simulink model "AcasXuClosedLoop_all_comp"  (Simulink 10.0)
settings: Solver=VariableStepAuto  StartTime=0.0  StopTime=20  RelTol=1e-3
libraries: neural x14040, simulink x2
(part 27 of 534)

== system AcasXuClosedLoop_all_comp/networks/Networks_m_6/AcasXu_4_6/Layer 3 ==
  "a{2} " : Inport  PortDimensions=50
      mask Display: e = 0.06;c1 = [120 230 180]/255;c2 = ([120 230 180]+0)/(256+128);c3 = ([120 230 180]+128)/(256+128);patch([0 1 1 0],[0 0 1 1],c1);patch([0 1 1 1-e 1-e 0+e],[0 0 1 1-e 0+e 0+e],c2);patch([0 0 1 1-e 0+e 0+e],[0 1 1 1-e 1-e 0+e],c3);plot([0 1 1 0 0],[0 0 1 1 0]);
  Delays 1 : Subsystem
      mask Display: e = 0.06;c1 = [120 150 230]/255;c2 = ([120 150 230]+0)/383;c3 = ([120 150 230]+128)/383;patch([0 1 1 0],[0 0 1 1],c1);patch([0 1 1 1-e 1-e 0+e],[0 0 1 1-e 0+e 0+e],c2);patch([0 0 1 1-e 0+e 0+e],[0 1 1 1-e 1-e 0+e],c3);plot([0 1 1 0 0],[0 0 1 1 0]);disp('0');
  "LW{3,2}" : Subsystem
      mask Display: e = 0.06;c1 = [120 150 230]/255;c2 = ([120 150 230]+0)/383;c3 = ([120 150 230]+128)/383;patch([0 1 1 0],[0 0 1 1],c1);patch([0 1 1 1-e 1-e 0+e],[0 0 1 1-e 0+e 0+e],c2);patch([0 0 1 1-e 0+e 0+e],[0 1 1 1-e 1-e 0+e],c3);plot([0 1 1 0 0],[0 0 1 1 0]);disp('W');
  "b{3}" : Constant  Value=[1.25236999999999998323119143606163561344146728515625;-1.82186000000000003495870259939692914485931396484375;0.70690799999999998082245156183489598333835601806640625;0.67740699999999998137667489572777412831783294677734375;0.003204180000000000048954174047821652493439614772796630859375;-1.4122500000000000053290705182007513940334320068359375;-0.1815900000000000014122036873231991194188594818115234375;-2.066549999999999887023705014144070446491241455078125;-0.5674580000000000179483095053001306951045989990234375;-0.235665999999999986602716717243310995399951934814453125;-0.3740229999999999943582906780648045241832733154296875;-0.033654700000000002668709697672966285608708858489990234375;0.60203799999999996206412333776825107634067535400390625;-0.89405599999999996185806594439782202243804931640625;-1.5173799999999999510436055061290971934795379638671875;-0.09026889999999999913971038267845869995653629302978515625;-0.420567999999999997395860873439232818782329559326171875;-0.931707999999999980644815877894870936870574951171875;-0.06573710000000000663877841589055606164038181304931640625;-0.339210000000000011510792319313623011112213134765625;-0.75268800000000002370370566495694220066070556640625;-1.114430000000000031690206014900468289852142333984375;-0.5772559999999999913455894784419797360897064208984375;0.357165000000000010249578963339445181190967559814453125;-1.9052100000000000701305680195218883454799652099609375;0.109812999999999993949728605002746917307376861572265625;2.244800000000000128608235172578133642673492431640625;-2.8195899999999998186694938340224325656890869140625;-0.2012519999999999864570554564124904572963714599609375;-1.2382999999999999563016217507538385689258575439453125;0.1436860000000000081588069633653503842651844024658203125;0.11354699999999999515676307737521710805594921112060546875;-0.571150999999999964273911245982162654399871826171875;-0.53546199999999999352695567722548730671405792236328125;-1.1326199999999999601385525238583795726299285888671875;0.90043499999999998539834678012994118034839630126953125;0.56335199999999996389732359602930955588817596435546875;-0.2246470000000000133422162207352812401950359344482421875;-1.126980000000000092796881290269084274768829345703125;-0.93247400000000002506794771761633455753326416015625;-1.440020000000000077733375292154960334300994873046875;-0.73937600000000003319655661471188068389892578125;-0.835104999999999986215470926254056394100189208984375;0.3871350000000000068922645368729718029499053955078125;-1.428290000000000059543481256696395576000213623046875;-0.748561000000000031917579690343700349330902099609375;-0.296891999999999989245935694270883686840534210205078125;-1.4761800000000000476774175695027224719524383544921875;0.352663000000000004252598273524199612438678741455078125;0.5822629999999999750315282653900794684886932373046875]
      mask Display: e = 0.06;c1 = [120 150 230]/255;c2 = ([120 150 230]+0)/383;c3 = ([120 150 230]+128)/383;patch([0 1 1 0],[0 0 1 1],c1);patch([0 1 1 1-e 1-e 0+e],[0 0 1 1-e 0+e 0+e],c2);patch([0 0 1 1-e 0+e 0+e],[0 1 1 1-e 1-e 0+e],c3);plot([0 1 1 0 0],[0 0 1 1 0]);disp('b');
  netsum : Sum  Inputs=2  SaturateOnIntegerOverflow=on  InputSameDT=on  OutDataTypeStr=Inherit: Same as first input
      mask Display: e = 0.06;c1 = [120 150 230]/255;c2 = ([120 150 230]+0)/383;c3 = ([120 150 230]+128)/383;patch([0 1 1 0],[0 0 1 1],c1);patch([0 1 1 1-e 1-e 0+e],[0 0 1 1-e 0+e 0+e],c2);patch([0 0 1 1-e 0+e 0+e],[0 1 1 1-e 1-e 0+e],c3);plot([0 1 1 0 0],[0 0 1 1 0]);disp('+');
  poslin : neural/Transfer Functions/poslin
      settings: SourceProductName=Deep Learning Toolbox  SourceProductBaseCode=NN
  "a{3}" : Outport  InitialOutput=[0;0;0;0;0;0;0;0;0;0;0;0;0;0;0;0;0;0;0;0;0;0;0;0;0;0;0;0;0;0;0;0;0;0;0;0;0;0;0;0;0;0;0;0;0;0;0;0;0;0]
      mask Display: e = 0.06;c1 = [120 230 180]/255;c2 = ([120 230 180]+0)/(256+128);c3 = ([120 230 180]+128)/(256+128);patch([0 1 1 0],[0 0 1 1],c1);patch([0 1 1 1-e 1-e 0+e],[0 0 1 1-e 0+e 0+e],c2);patch([0 0 1 1-e 0+e 0+e],[0 1 1 1-e 1-e 0+e],c3);plot([0 1 1 0 0],[0 0 1 1 0]);
  wires:
  poslin/1 -> "a{3}"/1
  netsum/1 -> poslin/1
  "b{3}"/1 -> netsum/2
  "LW{3,2}"/1("lz{3,2}") -> netsum/1
  Delays 1/1("pd{3,2}") -> "LW{3,2}"/1("ad{3,2}")
  "a{2} "/1 -> Delays 1/1("p{3}")

== system AcasXuClosedLoop_all_comp/networks/Networks_m_6/AcasXu_4_6/Layer 4 ==
  "a{3} " : Inport  PortDimensions=50
      mask Display: e = 0.06;c1 = [120 230 180]/255;c2 = ([120 230 180]+0)/(256+128);c3 = ([120 230 180]+128)/(256+128);patch([0 1 1 0],[0 0 1 1],c1);patch([0 1 1 1-e 1-e 0+e],[0 0 1 1-e 0+e 0+e],c2);patch([0 0 1 1-e 0+e 0+e],[0 1 1 1-e 1-e 0+e],c3);plot([0 1 1 0 0],[0 0 1 1 0]);
  Delays 1 : Subsystem
      mask Display: e = 0.06;c1 = [120 150 230]/255;c2 = ([120 150 230]+0)/383;c3 = ([120 150 230]+128)/383;patch([0 1 1 0],[0 0 1 1],c1);patch([0 1 1 1-e 1-e 0+e],[0 0 1 1-e 0+e 0+e],c2);patch([0 0 1 1-e 0+e 0+e],[0 1 1 1-e 1-e 0+e],c3);plot([0 1 1 0 0],[0 0 1 1 0]);disp('0');
  "LW{4,3}" : Subsystem
      mask Display: e = 0.06;c1 = [120 150 230]/255;c2 = ([120 150 230]+0)/383;c3 = ([120 150 230]+128)/383;patch([0 1 1 0],[0 0 1 1],c1);patch([0 1 1 1-e 1-e 0+e],[0 0 1 1-e 0+e 0+e],c2);patch([0 0 1 1-e 0+e 0+e],[0 1 1 1-e 1-e 0+e],c3);plot([0 1 1 0 0],[0 0 1 1 0]);disp('W');
  "b{4}" : Constant  Value=[-3.334090000000000220126139538479037582874298095703125;-0.76609099999999996644106659005046822130680084228515625;-0.047776800000000001211741817996880854479968547821044921875;1.966110000000000024300561562995426356792449951171875;-1.510219999999999895834434937569312751293182373046875;-2.430120000000000057838178690872155129909515380859375;-1.6310599999999999543121020906255580484867095947265625;0.365655000000000007798206524967099539935588836669921875;1.6739200000000000745359329812345094978809356689453125;-0.32896599999999998065192130525247193872928619384765625;2.846200000000000063238303482648916542530059814453125;-0.67345800000000000107291953099775128066539764404296875;-0.61934500000000003439026841078884899616241455078125;-2.510380000000000055848659030743874609470367431640625;-0.0987428999999999945647033428031136281788349151611328125;-3.1409099999999998686917024315334856510162353515625;0.2509770000000000056417093219351954758167266845703125;0.9941429999999999989057641869294457137584686279296875;-1.0512200000000000432720526077901013195514678955078125;-1.0510900000000000797939492258592508733272552490234375;-0.1089090000000000058033577943206182681024074554443359375;-2.9356700000000000017053025658242404460906982421875;-0.15021899999999999142374917937559075653553009033203125;-2.948770000000000113260512080159969627857208251953125;0.08955470000000000097006846999647677876055240631103515625;1.2601299999999999723598875789321027696132659912109375;2.14989000000000007872813512221910059452056884765625;0.5562270000000000269579913947382010519504547119140625;-1.414260000000000072617467594682238996028900146484375;-2.142910000000000092512664195965044200420379638671875;0.08335019999999999928963489992383983917534351348876953125;-0.452707000000000026052049406644073314964771270751953125;0.73034699999999996844479710489395074546337127685546875;0.386303000000000007485567721232655458152294158935546875;0.2329029999999999989146459711264469660818576812744140625;-1.114360000000000017195134205394424498081207275390625;-4.6393000000000004234834705130197107791900634765625;0.265996999999999983455012397826067171990871429443359375;0.037446699999999999430855268656159751117229461669921875;-0.84933800000000003738165332833887077867984771728515625;0.08166610000000000535447242100417497567832469940185546875;-0.407220999999999999641175918441149406135082244873046875;-1.1031500000000000749622586226905696094036102294921875;-0.765932999999999974960473991814069449901580810546875;1.071849999999999969446662362315692007541656494140625;0.6187460000000000182041048901737667620182037353515625;-1.0859799999999999453592636200482957065105438232421875;0.0642923999999999995491606341602164320647716522216796875;0.260197999999999984854781587273464538156986236572265625;-4.03394999999999992468247000942938029766082763671875]
      mask Display: e = 0.06;c1 = [120 150 230]/255;c2 = ([120 150 230]+0)/383;c3 = ([120 150 230]+128)/383;patch([0 1 1 0],[0 0 1 1],c1);patch([0 1 1 1-e 1-e 0+e],[0 0 1 1-e 0+e 0+e],c2);patch([0 0 1 1-e 0+e 0+e],[0 1 1 1-e 1-e 0+e],c3);plot([0 1 1 0 0],[0 0 1 1 0]);disp('b');
  netsum : Sum  Inputs=2  SaturateOnIntegerOverflow=on  InputSameDT=on  OutDataTypeStr=Inherit: Same as first input
      mask Display: e = 0.06;c1 = [120 150 230]/255;c2 = ([120 150 230]+0)/383;c3 = ([120 150 230]+128)/383;patch([0 1 1 0],[0 0 1 1],c1);patch([0 1 1 1-e 1-e 0+e],[0 0 1 1-e 0+e 0+e],c2);patch([0 0 1 1-e 0+e 0+e],[0 1 1 1-e 1-e 0+e],c3);plot([0 1 1 0 0],[0 0 1 1 0]);disp('+');
  poslin : neural/Transfer Functions/poslin
      settings: SourceProductName=Deep Learning Toolbox  SourceProductBaseCode=NN
  "a{4}" : Outport  InitialOutput=[0;0;0;0;0;0;0;0;0;0;0;0;0;0;0;0;0;0;0;0;0;0;0;0;0;0;0;0;0;0;0;0;0;0;0;0;0;0;0;0;0;0;0;0;0;0;0;0;0;0]
      mask Display: e = 0.06;c1 = [120 230 180]/255;c2 = ([120 230 180]+0)/(256+128);c3 = ([120 230 180]+128)/(256+128);patch([0 1 1 0],[0 0 1 1],c1);patch([0 1 1 1-e 1-e 0+e],[0 0 1 1-e 0+e 0+e],c2);patch([0 0 1 1-e 0+e 0+e],[0 1 1 1-e 1-e 0+e],c3);plot([0 1 1 0 0],[0 0 1 1 0]);
  wires:
  poslin/1 -> "a{4}"/1
  netsum/1 -> poslin/1
  "b{4}"/1 -> netsum/2
  "LW{4,3}"/1("lz{4,3}") -> netsum/1
  Delays 1/1("pd{4,3}") -> "LW{4,3}"/1("ad{4,3}")
  "a{3} "/1 -> Delays 1/1("p{4}")

== system AcasXuClosedLoop_all_comp/networks/Networks_m_6/AcasXu_4_6/Layer 5 ==
  "a{4} " : Inport  PortDimensions=50
      mask Display: e = 0.06;c1 = [120 230 180]/255;c2 = ([120 230 180]+0)/(256+128);c3 = ([120 230 180]+128)/(256+128);patch([0 1 1 0],[0 0 1 1],c1);patch([0 1 1 1-e 1-e 0+e],[0 0 1 1-e 0+e 0+e],c2);patch([0 0 1 1-e 0+e 0+e],[0 1 1 1-e 1-e 0+e],c3);plot([0 1 1 0 0],[0 0 1 1 0]);
  Delays 1 : Subsystem
      mask Display: e = 0.06;c1 = [120 150 230]/255;c2 = ([120 150 230]+0)/383;c3 = ([120 150 230]+128)/383;patch([0 1 1 0],[0 0 1 1],c1);patch([0 1 1 1-e 1-e 0+e],[0 0 1 1-e 0+e 0+e],c2);patch([0 0 1 1-e 0+e 0+e],[0 1 1 1-e 1-e 0+e],c3);plot([0 1 1 0 0],[0 0 1 1 0]);disp('0');
  "LW{5,4}" : Subsystem
      mask Display: e = 0.06;c1 = [120 150 230]/255;c2 = ([120 150 230]+0)/383;c3 = ([120 150 230]+128)/383;patch([0 1 1 0],[0 0 1 1],c1);patch([0 1 1 1-e 1-e 0+e],[0 0 1 1-e 0+e 0+e],c2);patch([0 0 1 1-e 0+e 0+e],[0 1 1 1-e 1-e 0+e],c3);plot([0 1 1 0 0],[0 0 1 1 0]);disp('W');
  "b{5}" : Constant  Value=[7.29755999999999982463805281440727412700653076171875;2.378270000000000106155084722558967769145965576171875;1.0495699999999998919264498908887617290019989013671875;0.049405499999999998028687997475572046823799610137939453125;0.315614000000000005652367462971596978604793548583984375;-2.0422799999999998732391759403981268405914306640625;1.452949999999999963762320476234890520572662353515625;0.069115800000000005010036829844466410577297210693359375;-1.1201499999999999790389892950770445168018341064453125;-0.5794470000000000453610482509247958660125732421875;-1.22961000000000009180212146020494401454925537109375;1.4459500000000000685673740008496679365634918212890625;0.731913000000000035782932172878645360469818115234375;-0.3770729999999999915161197350244037806987762451171875;-1.46943000000000001392663762089796364307403564453125;-1.7672600000000000530775423612794838845729827880859375;-0.238638999999999990020427276249392889440059661865234375;2.322200000000000041922021409845910966396331787109375;-3.855469999999999952677853798377327620983123779296875;-2.9819300000000001915623215609230101108551025390625;1.7324800000000000199662508748588152229785919189453125;-0.6646840000000000525659515915322117507457733154296875;1.272510000000000029984903449076227843761444091796875;1.482979999999999964899188853451050817966461181640625;-2.415369999999999794937366459635086357593536376953125;0.5639279999999999848370180188794620335102081298828125;-0.61243700000000000915889586394769139587879180908203125;-3.99744000000000010430767360958270728588104248046875;0.0025131699999999999005362294468568506999872624874114990234375;0.876219999999999998863131622783839702606201171875;3.470610000000000194830818145419470965862274169921875;3.96886999999999989796606314484961330890655517578125;0.55980799999999997229593873271369375288486480712890625;-1.7959300000000000263611354966997168958187103271484375;0.06655750000000000554667423102728207595646381378173828125;-2.10503999999999980019538270425982773303985595703125;-1.697070000000000078443918027915060520172119140625;1.6019900000000000250821585723315365612506866455078125;-2.245880000000000098481223176349885761737823486328125;-1.4869499999999999939603867460391484200954437255859375;-3.01977999999999990876631272840313613414764404296875;-2.20718000000000014182433005771599709987640380859375;1.0999499999999999833022457096376456320285797119140625;-2.7629999999999999005240169935859739780426025390625;-0.11246299999999999352784385564518743194639682769775390625;0.48169099999999998029664993737242184579372406005859375;0.66145299999999995765875837605562992393970489501953125;-0.61481799999999997563548959078616462647914886474609375;-1.536399999999999987920773492078296840190887451171875;1.80051000000000005485389920067973434925079345703125]
      mask Display: e = 0.06;c1 = [120 150 230]/255;c2 = ([120 150 230]+0)/383;c3 = ([120 150 230]+128)/383;patch([0 1 1 0],[0 0 1 1],c1);patch([0 1 1 1-e 1-e 0+e],[0 0 1 1-e 0+e 0+e],c2);patch([0 0 1 1-e 0+e 0+e],[0 1 1 1-e 1-e 0+e],c3);plot([0 1 1 0 0],[0 0 1 1 0]);disp('b');
  netsum : Sum  Inputs=2  SaturateOnIntegerOverflow=on  InputSameDT=on  OutDataTypeStr=Inherit: Same as first input
      mask Display: e = 0.06;c1 = [120 150 230]/255;c2 = ([120 150 230]+0)/383;c3 = ([120 150 230]+128)/383;patch([0 1 1 0],[0 0 1 1],c1);patch([0 1 1 1-e 1-e 0+e],[0 0 1 1-e 0+e 0+e],c2);patch([0 0 1 1-e 0+e 0+e],[0 1 1 1-e 1-e 0+e],c3);plot([0 1 1 0 0],[0 0 1 1 0]);disp('+');
  poslin : neural/Transfer Functions/poslin
      settings: SourceProductName=Deep Learning Toolbox  SourceProductBaseCode=NN
  "a{5}" : Outport  InitialOutput=[0;0;0;0;0;0;0;0;0;0;0;0;0;0;0;0;0;0;0;0;0;0;0;0;0;0;0;0;0;0;0;0;0;0;0;0;0;0;0;0;0;0;0;0;0;0;0;0;0;0]
      mask Display: e = 0.06;c1 = [120 230 180]/255;c2 = ([120 230 180]+0)/(256+128);c3 = ([120 230 180]+128)/(256+128);patch([0 1 1 0],[0 0 1 1],c1);patch([0 1 1 1-e 1-e 0+e],[0 0 1 1-e 0+e 0+e],c2);patch([0 0 1 1-e 0+e 0+e],[0 1 1 1-e 1-e 0+e],c3);plot([0 1 1 0 0],[0 0 1 1 0]);
  wires:
  poslin/1 -> "a{5}"/1
  netsum/1 -> poslin/1
  "b{5}"/1 -> netsum/2
  "LW{5,4}"/1("lz{5,4}") -> netsum/1
  Delays 1/1("pd{5,4}") -> "LW{5,4}"/1("ad{5,4}")
  "a{4} "/1 -> Delays 1/1("p{5}")

== system AcasXuClosedLoop_all_comp/networks/Networks_m_6/AcasXu_4_6/Layer 6 ==
  "a{5} " : Inport  PortDimensions=50
      mask Display: e = 0.06;c1 = [120 230 180]/255;c2 = ([120 230 180]+0)/(256+128);c3 = ([120 230 180]+128)/(256+128);patch([0 1 1 0],[0 0 1 1],c1);patch([0 1 1 1-e 1-e 0+e],[0 0 1 1-e 0+e 0+e],c2);patch([0 0 1 1-e 0+e 0+e],[0 1 1 1-e 1-e 0+e],c3);plot([0 1 1 0 0],[0 0 1 1 0]);
  Delays 1 : Subsystem
      mask Display: e = 0.06;c1 = [120 150 230]/255;c2 = ([120 150 230]+0)/383;c3 = ([120 150 230]+128)/383;patch([0 1 1 0],[0 0 1 1],c1);patch([0 1 1 1-e 1-e 0+e],[0 0 1 1-e 0+e 0+e],c2);patch([0 0 1 1-e 0+e 0+e],[0 1 1 1-e 1-e 0+e],c3);plot([0 1 1 0 0],[0 0 1 1 0]);disp('0');
  "LW{6,5}" : Subsystem
      mask Display: e = 0.06;c1 = [120 150 230]/255;c2 = ([120 150 230]+0)/383;c3 = ([120 150 230]+128)/383;patch([0 1 1 0],[0 0 1 1],c1);patch([0 1 1 1-e 1-e 0+e],[0 0 1 1-e 0+e 0+e],c2);patch([0 0 1 1-e 0+e 0+e],[0 1 1 1-e 1-e 0+e],c3);plot([0 1 1 0 0],[0 0 1 1 0]);disp('W');
  "b{6}" : Constant  Value=[-5.06167000000000033566038837307132780551910400390625;-4.11592000000000002302158463862724602222442626953125;0.167069999999999996287414205653476528823375701904296875;-0.380635000000000001119104808822157792747020721435546875;-5.86861999999999994770405464805662631988525390625;2.603940000000000143387524076388217508792877197265625;0.52917899999999995497290683488245122134685516357421875;0.329865000000000019308998844280722551047801971435546875;0.207413999999999987267074175179004669189453125;-1.9459900000000001085709300241433084011077880859375;-3.344500000000000028421709430404007434844970703125;3.069570000000000131734623209922574460506439208984375;0.454330999999999984861887014631065540015697479248046875;0.630163000000000028677504815277643501758575439453125;1.2560500000000001108446667785756289958953857421875;-1.495530000000000026005864128819666802883148193359375;-5.91870000000000029416469260468147695064544677734375;2.849660000000000081854523159563541412353515625;0.93860200000000004738609504784108139574527740478515625;-1.626160000000000049880100050359033048152923583984375;0.48533199999999998563993131028837524354457855224609375;-4.6092700000000004223466021358035504817962646484375;0.73320499999999999563016217507538385689258575439453125;-5.71459000000000028052227207808755338191986083984375;2.15219000000000004746425474877469241619110107421875;-0.03185559999999999780673221039251075126230716705322265625;-3.457199999999999828759200681815855205059051513671875;-6.134850000000000136424205265939235687255859375;0.70433000000000001161737372967763803899288177490234375;-0.96110799999999996234834043207229115068912506103515625;-6.8291100000000000136424205265939235687255859375;-4.57237000000000026744828574010170996189117431640625;0.497414999999999996038724248137441463768482208251953125;-0.0376678000000000012814638239433406852185726165771484375;-3.795030000000000125481847135233692824840545654296875;-1.38759999999999994457766661071218550205230712890625;-4.8285099999999996356336851022206246852874755859375;-0.6228949999999999764810354463406838476657867431640625;1.3975400000000000044764192352886311709880828857421875;-0.0350444000000000033256952747251489199697971343994140625;-4.83635999999999999232613845379091799259185791015625;-0.87470499999999995477395486886962316930294036865234375;-3.450790000000000024016344468691386282444000244140625;-2.68121000000000009322320693172514438629150390625;0.473156999999999994255261981379589997231960296630859375;-0.29870600000000002705036195038701407611370086669921875;-1.1608000000000000540012479177676141262054443359375;-4.3208999999999999630517777404747903347015380859375;-3.161319999999999907913661445491015911102294921875;-1.5834900000000000641620090391370467841625213623046875]
      mask Display: e = 0.06;c1 = [120 150 230]/255;c2 = ([120 150 230]+0)/383;c3 = ([120 150 230]+128)/383;patch([0 1 1 0],[0 0 1 1],c1);patch([0 1 1 1-e 1-e 0+e],[0 0 1 1-e 0+e 0+e],c2);patch([0 0 1 1-e 0+e 0+e],[0 1 1 1-e 1-e 0+e],c3);plot([0 1 1 0 0],[0 0 1 1 0]);disp('b');
  netsum : Sum  Inputs=2  SaturateOnIntegerOverflow=on  InputSameDT=on  OutDataTypeStr=Inherit: Same as first input
      mask Display: e = 0.06;c1 = [120 150 230]/255;c2 = ([120 150 230]+0)/383;c3 = ([120 150 230]+128)/383;patch([0 1 1 0],[0 0 1 1],c1);patch([0 1 1 1-e 1-e 0+e],[0 0 1 1-e 0+e 0+e],c2);patch([0 0 1 1-e 0+e 0+e],[0 1 1 1-e 1-e 0+e],c3);plot([0 1 1 0 0],[0 0 1 1 0]);disp('+');
  poslin : neural/Transfer Functions/poslin
      settings: SourceProductName=Deep Learning Toolbox  SourceProductBaseCode=NN
  "a{6}" : Outport  InitialOutput=[0;0;0;0;0;0;0;0;0;0;0;0;0;0;0;0;0;0;0;0;0;0;0;0;0;0;0;0;0;0;0;0;0;0;0;0;0;0;0;0;0;0;0;0;0;0;0;0;0;0]
      mask Display: e = 0.06;c1 = [120 230 180]/255;c2 = ([120 230 180]+0)/(256+128);c3 = ([120 230 180]+128)/(256+128);patch([0 1 1 0],[0 0 1 1],c1);patch([0 1 1 1-e 1-e 0+e],[0 0 1 1-e 0+e 0+e],c2);patch([0 0 1 1-e 0+e 0+e],[0 1 1 1-e 1-e 0+e],c3);plot([0 1 1 0 0],[0 0 1 1 0]);
  wires:
  poslin/1 -> "a{6}"/1
  netsum/1 -> poslin/1
  "b{6}"/1 -> netsum/2
  "LW{6,5}"/1("lz{6,5}") -> netsum/1
  Delays 1/1("pd{6,5}") -> "LW{6,5}"/1("ad{6,5}")
  "a{5} "/1 -> Delays 1/1("p{6}")

== system AcasXuClosedLoop_all_comp/networks/Networks_m_6/AcasXu_4_6/Layer 7 ==
  "a{6} " : Inport  PortDimensions=50
      mask Display: e = 0.06;c1 = [120 230 180]/255;c2 = ([120 230 180]+0)/(256+128);c3 = ([120 230 180]+128)/(256+128);patch([0 1 1 0],[0 0 1 1],c1);patch([0 1 1 1-e 1-e 0+e],[0 0 1 1-e 0+e 0+e],c2);patch([0 0 1 1-e 0+e 0+e],[0 1 1 1-e 1-e 0+e],c3);plot([0 1 1 0 0],[0 0 1 1 0]);
  Delays 1 : Subsystem
      mask Display: e = 0.06;c1 = [120 150 230]/255;c2 = ([120 150 230]+0)/383;c3 = ([120 150 230]+128)/383;patch([0 1 1 0],[0 0 1 1],c1);patch([0 1 1 1-e 1-e 0+e],[0 0 1 1-e 0+e 0+e],c2);patch([0 0 1 1-e 0+e 0+e],[0 1 1 1-e 1-e 0+e],c3);plot([0 1 1 0 0],[0 0 1 1 0]);disp('0');
  "LW{7,6}" : Subsystem
      mask Display: e = 0.06;c1 = [120 150 230]/255;c2 = ([120 150 230]+0)/383;c3 = ([120 150 230]+128)/383;patch([0 1 1 0],[0 0 1 1],c1);patch([0 1 1 1-e 1-e 0+e],[0 0 1 1-e 0+e 0+e],c2);patch([0 0 1 1-e 0+e 0+e],[0 1 1 1-e 1-e 0+e],c3);plot([0 1 1 0 0],[0 0 1 1 0]);disp('W');
  "b{7}" : Constant  Value=[-0.01678090000000000137081457296517328359186649322509765625;-0.017631000000000000837996338987068156711757183074951171875;0.0206924999999999990885068967827464803121984004974365234375;-0.0168419999999999993323118729904308565892279148101806640625;0.0202648999999999988863574884589979774318635463714599609375]
      mask Display: e = 0.06;c1 = [120 150 230]/255;c2 = ([120 150 230]+0)/383;c3 = ([120 150 230]+128)/383;patch([0 1 1 0],[0 0 1 1],c1);patch([0 1 1 1-e 1-e 0+e],[0 0 1 1-e 0+e 0+e],c2);patch([0 0 1 1-e 0+e 0+e],[0 1 1 1-e 1-e 0+e],c3);plot([0 1 1 0 0],[0 0 1 1 0]);disp('b');
  netsum : Sum  Inputs=2  SaturateOnIntegerOverflow=on  InputSameDT=on  OutDataTypeStr=Inherit: Same as first input
      mask Display: e = 0.06;c1 = [120 150 230]/255;c2 = ([120 150 230]+0)/383;c3 = ([120 150 230]+128)/383;patch([0 1 1 0],[0 0 1 1],c1);patch([0 1 1 1-e 1-e 0+e],[0 0 1 1-e 0+e 0+e],c2);patch([0 0 1 1-e 0+e 0+e],[0 1 1 1-e 1-e 0+e],c3);plot([0 1 1 0 0],[0 0 1 1 0]);disp('+');
  purelin : neural/Transfer Functions/purelin
      settings: SourceProductName=Deep Learning Toolbox
  "a{7}" : Outport  InitialOutput=[0;0;0;0;0]
      mask Display: e = 0.06;c1 = [120 230 180]/255;c2 = ([120 230 180]+0)/(256+128);c3 = ([120 230 180]+128)/(256+128);patch([0 1 1 0],[0 0 1 1],c1);patch([0 1 1 1-e 1-e 0+e],[0 0 1 1-e 0+e 0+e],c2);patch([0 0 1 1-e 0+e 0+e],[0 1 1 1-e 1-e 0+e],c3);plot([0 1 1 0 0],[0 0 1 1 0]);
  wires:
  purelin/1 -> "a{7}"/1
  netsum/1 -> purelin/1
  "b{7}"/1 -> netsum/2
  "LW{7,6}"/1("lz{7,6}") -> netsum/1
  Delays 1/1("pd{7,6}") -> "LW{7,6}"/1("ad{7,6}")
  "a{6} "/1 -> Delays 1/1("p{7}")

== system AcasXuClosedLoop_all_comp/networks/Networks_m_6/AcasXu_4_6/Process Input 1 ==
  x : Inport  PortDimensions=5
      mask Display: e = 0.06;c1 = [120 230 180]/255;c2 = ([120 230 180]+0)/(256+128);c3 = ([120 230 180]+128)/(256+128);patch([0 1 1 0],[0 0 1 1],c1);patch([0 1 1 1-e 1-e 0+e],[0 0 1 1-e 0+e 0+e],c2);patch([0 0 1 1-e 0+e 0+e],[0 1 1 1-e 1-e 0+e],c3);plot([0 1 1 0 0],[0 0 1 1 0]);
  p : Outport  PortDimensions=5
      mask Display: e = 0.06;c1 = [120 230 180]/255;c2 = ([120 230 180]+0)/(256+128);c3 = ([120 230 180]+128)/(256+128);patch([0 1 1 0],[0 0 1 1],c1);patch([0 1 1 1-e 1-e 0+e],[0 0 1 1-e 0+e 0+e],c2);patch([0 0 1 1-e 0+e 0+e],[0 1 1 1-e 1-e 0+e],c3);plot([0 1 1 0 0],[0 0 1 1 0]);
  wires:
  x/1 -> p/1

== system AcasXuClosedLoop_all_comp/networks/Networks_m_6/AcasXu_4_6/Process Output 1 ==
  a : Inport  PortDimensions=5
      mask Display: e = 0.06;c1 = [120 230 180]/255;c2 = ([120 230 180]+0)/(256+128);c3 = ([120 230 180]+128)/(256+128);patch([0 1 1 0],[0 0 1 1],c1);patch([0 1 1 1-e 1-e 0+e],[0 0 1 1-e 0+e 0+e],c2);patch([0 0 1 1-e 0+e 0+e],[0 1 1 1-e 1-e 0+e],c3);plot([0 1 1 0 0],[0 0 1 1 0]);
  y : Outport  PortDimensions=5
      mask Display: e = 0.06;c1 = [120 230 180]/255;c2 = ([120 230 180]+0)/(256+128);c3 = ([120 230 180]+128)/(256+128);patch([0 1 1 0],[0 0 1 1],c1);patch([0 1 1 1-e 1-e 0+e],[0 0 1 1-e 0+e 0+e],c2);patch([0 0 1 1-e 0+e 0+e],[0 1 1 1-e 1-e 0+e],c3);plot([0 1 1 0 0],[0 0 1 1 0]);
  wires:
  a/1 -> y/1

== system AcasXuClosedLoop_all_comp/networks/Networks_m_6/AcasXu_5_6/Layer 1 ==
  "p{1}" : Inport  PortDimensions=5
      mask Display: e = 0.06;c1 = [120 230 180]/255;c2 = ([120 230 180]+0)/(256+128);c3 = ([120 230 180]+128)/(256+128);patch([0 1 1 0],[0 0 1 1],c1);patch([0 1 1 1-e 1-e 0+e],[0 0 1 1-e 0+e 0+e],c2);patch([0 0 1 1-e 0+e 0+e],[0 1 1 1-e 1-e 0+e],c3);plot([0 1 1 0 0],[0 0 1 1 0]);
  Delays 1 : Subsystem
      mask Display: e = 0.06;c1 = [120 150 230]/255;c2 = ([120 150 230]+0)/383;c3 = ([120 150 230]+128)/383;patch([0 1 1 0],[0 0 1 1],c1);patch([0 1 1 1-e 1-e 0+e],[0 0 1 1-e 0+e 0+e],c2);patch([0 0 1 1-e 0+e 0+e],[0 1 1 1-e 1-e 0+e],c3);plot([0 1 1 0 0],[0 0 1 1 0]);disp('0');
  "IW{1,1}" : Subsystem
      mask Display: e = 0.06;c1 = [120 150 230]/255;c2 = ([120 150 230]+0)/383;c3 = ([120 150 230]+128)/383;patch([0 1 1 0],[0 0 1 1],c1);patch([0 1 1 1-e 1-e 0+e],[0 0 1 1-e 0+e 0+e],c2);patch([0 0 1 1-e 0+e 0+e],[0 1 1 1-e 1-e 0+e],c3);plot([0 1 1 0 0],[0 0 1 1 0]);disp('W');
  "b{1}" : Constant  Value=[-0.56657699999999999729283217675401829183101654052734375;-0.0256695000000000013440359936112145078368484973907470703125;0.27909200000000000674305056236335076391696929931640625;-0.2456480000000000052384763193913386203348636627197265625;-0.2204250000000000098143715376863838173449039459228515625;-0.364111999999999991217691786005161702632904052734375;0.09129030000000000477644590546333347447216510772705078125;-0.40700700000000000766675611885148100554943084716796875;-0.47744900000000001227817847393453121185302734375;-0.48022799999999998821209601373993791639804840087890625;-0.40090599999999998459543348872102797031402587890625;-0.2041719999999999923812055158123257569968700408935546875;0.457562999999999997502442283803247846662998199462890625;-0.1527179999999999926441063280435628257691860198974609375;-0.609536999999999995480948200565762817859649658203125;-0.1170960000000000056363802514169947244226932525634765625;-0.014380600000000000326938476291616098023951053619384765625;-0.147390999999999994241051126664387993514537811279296875;-0.68103499999999994596322494544438086450099945068359375;-0.214088000000000000522248910783673636615276336669921875;0.06183729999999999782378523605075315572321414947509765625;-0.77008200000000004425970701049664057791233062744140625;-0.001479349999999999935862415867404706659726798534393310546875;0.1176480000000000025739410602909629233181476593017578125;0.162706999999999990524912618639064021408557891845703125;-0.2142799999999999982502885131907532922923564910888671875;-0.348189000000000026258106800014502368867397308349609375;0.046726099999999999579092246904110652394592761993408203125;0.355069999999999996731503415503539144992828369140625;-0.0200982000000000000927702359376780805177986621856689453125;-0.153915999999999997260857753644813783466815948486328125;-0.626692999999999944549244901281781494617462158203125;-0.194659999999999999698019337301957421004772186279296875;-0.0228748000000000006881606395836570300161838531494140625;0.1363379999999999869775280103567638434469699859619140625;0.00823214000000000063750338341606038738973438739776611328125;-0.4146389999999999798063754496979527175426483154296875;-0.27468700000000001448796638214844278991222381591796875;-0.374551999999999996049382389173842966556549072265625;-0.156185999999999991505461593988002277910709381103515625;-0.1457219999999999904272129924720502458512783050537109375;-0.02085700000000000053912430075797601602971553802490234375;-0.11877000000000000057287508070658077485859394073486328125;-0.2220000000000000028865798640254070051014423370361328125;0.08706640000000000212398987287087948061525821685791015625;-0.47836400000000001142552719102241098880767822265625;-0.2637140000000000039648284655413590371608734130859375;0.12048300000000000675726141707855276763439178466796875;-0.367466000000000014846790463707293383777141571044921875;0.005403169999999999674467066057559350156225264072418212890625]
      mask Display: e = 0.06;c1 = [120 150 230]/255;c2 = ([120 150 230]+0)/383;c3 = ([120 150 230]+128)/383;patch([0 1 1 0],[0 0 1 1],c1);patch([0 1 1 1-e 1-e 0+e],[0 0 1 1-e 0+e 0+e],c2);patch([0 0 1 1-e 0+e 0+e],[0 1 1 1-e 1-e 0+e],c3);plot([0 1 1 0 0],[0 0 1 1 0]);disp('b');
  netsum : Sum  Inputs=2  SaturateOnIntegerOverflow=on  InputSameDT=on  OutDataTypeStr=Inherit: Same as first input
      mask Display: e = 0.06;c1 = [120 150 230]/255;c2 = ([120 150 230]+0)/383;c3 = ([120 150 230]+128)/383;patch([0 1 1 0],[0 0 1 1],c1);patch([0 1 1 1-e 1-e 0+e],[0 0 1 1-e 0+e 0+e],c2);patch([0 0 1 1-e 0+e 0+e],[0 1 1 1-e 1-e 0+e],c3);plot([0 1 1 0 0],[0 0 1 1 0]);disp('+');
  poslin : neural/Transfer Functions/poslin
      settings: SourceProductName=Deep Learning Toolbox  SourceProductBaseCode=NN
  "a{1}" : Outport  InitialOutput=[0;0;0;0;0;0;0;0;0;0;0;0;0;0;0;0;0;0;0;0;0;0;0;0;0;0;0;0;0;0;0;0;0;0;0;0;0;0;0;0;0;0;0;0;0;0;0;0;0;0]
      mask Display: e = 0.06;c1 = [120 230 180]/255;c2 = ([120 230 180]+0)/(256+128);c3 = ([120 230 180]+128)/(256+128);patch([0 1 1 0],[0 0 1 1],c1);patch([0 1 1 1-e 1-e 0+e],[0 0 1 1-e 0+e 0+e],c2);patch([0 0 1 1-e 0+e 0+e],[0 1 1 1-e 1-e 0+e],c3);plot([0 1 1 0 0],[0 0 1 1 0]);
  wires:
  poslin/1 -> "a{1}"/1
  netsum/1 -> poslin/1
  "b{1}"/1 -> netsum/2
  "IW{1,1}"/1("iz{1,1}") -> netsum/1
  Delays 1/1("pd{1,1}") -> "IW{1,1}"/1("pd{1,1}")
  "p{1}"/1 -> Delays 1/1("p{1}")

== system AcasXuClosedLoop_all_comp/networks/Networks_m_6/AcasXu_5_6/Layer 2 ==
  "a{1} " : Inport  PortDimensions=50
      mask Display: e = 0.06;c1 = [120 230 180]/255;c2 = ([120 230 180]+0)/(256+128);c3 = ([120 230 180]+128)/(256+128);patch([0 1 1 0],[0 0 1 1],c1);patch([0 1 1 1-e 1-e 0+e],[0 0 1 1-e 0+e 0+e],c2);patch([0 0 1 1-e 0+e 0+e],[0 1 1 1-e 1-e 0+e],c3);plot([0 1 1 0 0],[0 0 1 1 0]);
  Delays 1 : Subsystem
      mask Display: e = 0.06;c1 = [120 150 230]/255;c2 = ([120 150 230]+0)/383;c3 = ([120 150 230]+128)/383;patch([0 1 1 0],[0 0 1 1],c1);patch([0 1 1 1-e 1-e 0+e],[0 0 1 1-e 0+e 0+e],c2);patch([0 0 1 1-e 0+e 0+e],[0 1 1 1-e 1-e 0+e],c3);plot([0 1 1 0 0],[0 0 1 1 0]);disp('0');
  "LW{2,1}" : Subsystem
      mask Display: e = 0.06;c1 = [120 150 230]/255;c2 = ([120 150 230]+0)/383;c3 = ([120 150 230]+128)/383;patch([0 1 1 0],[0 0 1 1],c1);patch([0 1 1 1-e 1-e 0+e],[0 0 1 1-e 0+e 0+e],c2);patch([0 0 1 1-e 0+e 0+e],[0 1 1 1-e 1-e 0+e],c3);plot([0 1 1 0 0],[0 0 1 1 0]);disp('W');
  "b{2}" : Constant  Value=[0.298895999999999995022648136000498197972774505615234375;-0.1462420000000000108730802139689330942928791046142578125;-1.1288400000000000655830945106572471559047698974609375;-0.006152059999999999993780530616049873060546815395355224609375;-0.63304400000000005110933898322400636970996856689453125;0.71372800000000002906830331994569860398769378662109375;-0.0065693200000000000204902761424818891100585460662841796875;1.4344500000000000028421709430404007434844970703125;-0.01100940000000000078272943682122786412946879863739013671875;-1.1263900000000000023447910280083306133747100830078125;-0.103578000000000003399946990612079389393329620361328125;-0.1366590000000000026947333253701799549162387847900390625;-0.2520350000000000090238927441532723605632781982421875;-0.005279240000000000147817313944642592105083167552947998046875;-1.0844899999999999540278849963215179741382598876953125;-0.046120500000000001772360036511599901132285594940185546875;0.25803100000000001035260766002465970814228057861328125;-0.0803864999999999996216359932077466510236263275146484375;-1.0545500000000000984101689027738757431507110595703125;-0.03852719999999999755946333834799588657915592193603515625;-0.03023030000000000161453073133088764734566211700439453125;0.2162550000000000027799984536613919772207736968994140625;-0.8384620000000000406004119213321246206760406494140625;-1.0271600000000000729727389625622890889644622802734375;-0.9097150000000000513722397954552434384822845458984375;0.73348500000000005361044941309955902397632598876953125;-0.327809999999999990283328088480629958212375640869140625;-0.0100184000000000002217337424781362642534077167510986328125;0.2412209999999999909814363263649283908307552337646484375;0.8387320000000000330686589222750626504421234130859375;-1.0668100000000000360245167030370794236660003662109375;1.65083000000000001961097950697876513004302978515625;1.1289000000000000145661260830820538103580474853515625;-0.0237482000000000005590639062802438274957239627838134765625;0.444234999999999990993870824240730144083499908447265625;-0.00807730000000000079862783053386010578833520412445068359375;-0.310157999999999989260146548986085690557956695556640625;-0.332133999999999984797938168412656523287296295166015625;-0.09401379999999999459436850202109781093895435333251953125;0.52406099999999999905497816143906675279140472412109375;0.75;-0.0206282999999999987206233953429546090774238109588623046875;-0.6995050000000000434141611549421213567256927490234375;-0.01425869999999999915285542329002055339515209197998046875;0.5958200000000000162714286489062942564487457275390625;1.3050200000000000688515910951537080109119415283203125;0.76638700000000004042277623739209957420825958251953125;0.5358739999999999614743728670873679220676422119140625;0.4908580000000000165272240337799303233623504638671875;-0.0251205000000000004678479825770409661345183849334716796875]
      mask Display: e = 0.06;c1 = [120 150 230]/255;c2 = ([120 150 230]+0)/383;c3 = ([120 150 230]+128)/383;patch([0 1 1 0],[0 0 1 1],c1);patch([0 1 1 1-e 1-e 0+e],[0 0 1 1-e 0+e 0+e],c2);patch([0 0 1 1-e 0+e 0+e],[0 1 1 1-e 1-e 0+e],c3);plot([0 1 1 0 0],[0 0 1 1 0]);disp('b');
  netsum : Sum  Inputs=2  SaturateOnIntegerOverflow=on  InputSameDT=on  OutDataTypeStr=Inherit: Same as first input
      mask Display: e = 0.06;c1 = [120 150 230]/255;c2 = ([120 150 230]+0)/383;c3 = ([120 150 230]+128)/383;patch([0 1 1 0],[0 0 1 1],c1);patch([0 1 1 1-e 1-e 0+e],[0 0 1 1-e 0+e 0+e],c2);patch([0 0 1 1-e 0+e 0+e],[0 1 1 1-e 1-e 0+e],c3);plot([0 1 1 0 0],[0 0 1 1 0]);disp('+');
  poslin : neural/Transfer Functions/poslin
      settings: SourceProductName=Deep Learning Toolbox  SourceProductBaseCode=NN
  "a{2}" : Outport  InitialOutput=[0;0;0;0;0;0;0;0;0;0;0;0;0;0;0;0;0;0;0;0;0;0;0;0;0;0;0;0;0;0;0;0;0;0;0;0;0;0;0;0;0;0;0;0;0;0;0;0;0;0]
      mask Display: e = 0.06;c1 = [120 230 180]/255;c2 = ([120 230 180]+0)/(256+128);c3 = ([120 230 180]+128)/(256+128);patch([0 1 1 0],[0 0 1 1],c1);patch([0 1 1 1-e 1-e 0+e],[0 0 1 1-e 0+e 0+e],c2);patch([0 0 1 1-e 0+e 0+e],[0 1 1 1-e 1-e 0+e],c3);plot([0 1 1 0 0],[0 0 1 1 0]);
  wires:
  poslin/1 -> "a{2}"/1
  netsum/1 -> poslin/1
  "b{2}"/1 -> netsum/2
  "LW{2,1}"/1("lz{2,1}") -> netsum/1
  Delays 1/1("pd{2,1}") -> "LW{2,1}"/1("ad{2,1}")
  "a{1} "/1 -> Delays 1/1("p{2}")

== system AcasXuClosedLoop_all_comp/networks/Networks_m_6/AcasXu_5_6/Layer 3 ==
  "a{2} " : Inport  PortDimensions=50
      mask Display: e = 0.06;c1 = [120 230 180]/255;c2 = ([120 230 180]+0)/(256+128);c3 = ([120 230 180]+128)/(256+128);patch([0 1 1 0],[0 0 1 1],c1);patch([0 1 1 1-e 1-e 0+e],[0 0 1 1-e 0+e 0+e],c2);patch([0 0 1 1-e 0+e 0+e],[0 1 1 1-e 1-e 0+e],c3);plot([0 1 1 0 0],[0 0 1 1 0]);
  Delays 1 : Subsystem
      mask Display: e = 0.06;c1 = [120 150 230]/255;c2 = ([120 150 230]+0)/383;c3 = ([120 150 230]+128)/383;patch([0 1 1 0],[0 0 1 1],c1);patch([0 1 1 1-e 1-e 0+e],[0 0 1 1-e 0+e 0+e],c2);patch([0 0 1 1-e 0+e 0+e],[0 1 1 1-e 1-e 0+e],c3);plot([0 1 1 0 0],[0 0 1 1 0]);disp('0');
  "LW{3,2}" : Subsystem
      mask Display: e = 0.06;c1 = [120 150 230]/255;c2 = ([120 150 230]+0)/383;c3 = ([120 150 230]+128)/383;patch([0 1 1 0],[0 0 1 1],c1);patch([0 1 1 1-e 1-e 0+e],[0 0 1 1-e 0+e 0+e],c2);patch([0 0 1 1-e 0+e 0+e],[0 1 1 1-e 1-e 0+e],c3);plot([0 1 1 0 0],[0 0 1 1 0]);disp('W');
  "b{3}" : Constant  Value=[0.260973999999999983767651201560511253774166107177734375;-1.3339399999999999035793507573544047772884368896484375;0.60432600000000002982147861985140480101108551025390625;-2.226269999999999971151964928139932453632354736328125;-1.5431500000000000216715534406830556690692901611328125;-0.31422099999999997255173411758732981979846954345703125;-1.7699700000000000432720526077901013195514678955078125;1.5100599999999999578648157694260589778423309326171875;-0.804540999999999950631490719388239085674285888671875;-0.47692699999999998983213345127296634018421173095703125;0.92996800000000001684696826487197540700435638427734375;0.68228900000000003434053041928564198315143585205078125;0.1483119999999999993889332472463138401508331298828125;0.320039000000000017909229654833325184881687164306640625;1.345909999999999939745976007543504238128662109375;0.0772802999999999962188468316526268608868122100830078125;-0.4335240000000000204494199351756833493709564208984375;-0.0306575000000000007227551890309769078157842159271240234375;-1.10827000000000008839151632855646312236785888671875;-0.51186100000000001042366193360066972672939300537109375;0.0081589000000000001799893567522303783334791660308837890625;-0.01066139999999999969648722952797470497898757457733154296875;-0.3428080000000000016058265828178264200687408447265625;-0.79123200000000004639133521777694113552570343017578125;0.61374099999999998100719267313252203166484832763671875;-0.20947999999999999953814722175593487918376922607421875;-3.047140000000000181756831807433627545833587646484375;-0.784653000000000044877879190607927739620208740234375;-1.6576299999999999368327507909270934760570526123046875;-0.0227861000000000001042277375518096960149705410003662109375;-2.956799999999999872812850298942066729068756103515625;0.10069899999999999684607843164485529996454715728759765625;-0.6515859999999999985220711096189916133880615234375;-0.1786290000000000099955599353052093647420406341552734375;-0.038327800000000002145217337101712473668158054351806640625;-0.57173499999999999321431687349104322493076324462890625;0.99304300000000000903099817151087336242198944091796875;0.9232650000000000023447910280083306133747100830078125;-0.1624170000000000058104632216782192699611186981201171875;-0.365653999999999979042542008755845017731189727783203125;0.759616999999999986670218277140520513057708740234375;-0.56354899999999996662580770134809426963329315185546875;-1.2203900000000000858335624798201024532318115234375;-0.79257100000000002548716793171479366719722747802734375;0.389272000000000006902922677909373305737972259521484375;0.1545970000000000121875842751251184381544589996337890625;-1.423550000000000093081098384573124349117279052734375;0.0256410000000000005970779426434091874398291110992431640625;-0.26619300000000001293898321819142438471317291259765625;-0.00986825000000000028543833963112774654291570186614990234375]
      mask Display: e = 0.06;c1 = [120 150 230]/255;c2 = ([120 150 230]+0)/383;c3 = ([120 150 230]+128)/383;patch([0 1 1 0],[0 0 1 1],c1);patch([0 1 1 1-e 1-e 0+e],[0 0 1 1-e 0+e 0+e],c2);patch([0 0 1 1-e 0+e 0+e],[0 1 1 1-e 1-e 0+e],c3);plot([0 1 1 0 0],[0 0 1 1 0]);disp('b');
  netsum : Sum  Inputs=2  SaturateOnIntegerOverflow=on  InputSameDT=on  OutDataTypeStr=Inherit: Same as first input
      mask Display: e = 0.06;c1 = [120 150 230]/255;c2 = ([120 150 230]+0)/383;c3 = ([120 150 230]+128)/383;patch([0 1 1 0],[0 0 1 1],c1);patch([0 1 1 1-e 1-e 0+e],[0 0 1 1-e 0+e 0+e],c2);patch([0 0 1 1-e 0+e 0+e],[0 1 1 1-e 1-e 0+e],c3);plot([0 1 1 0 0],[0 0 1 1 0]);disp('+');
  poslin : neural/Transfer Functions/poslin
      settings: SourceProductName=Deep Learning Toolbox  SourceProductBaseCode=NN
  "a{3}" : Outport  InitialOutput=[0;0;0;0;0;0;0;0;0;0;0;0;0;0;0;0;0;0;0;0;0;0;0;0;0;0;0;0;0;0;0;0;0;0;0;0;0;0;0;0;0;0;0;0;0;0;0;0;0;0]
      mask Display: e = 0.06;c1 = [120 230 180]/255;c2 = ([120 230 180]+0)/(256+128);c3 = ([120 230 180]+128)/(256+128);patch([0 1 1 0],[0 0 1 1],c1);patch([0 1 1 1-e 1-e 0+e],[0 0 1 1-e 0+e 0+e],c2);patch([0 0 1 1-e 0+e 0+e],[0 1 1 1-e 1-e 0+e],c3);plot([0 1 1 0 0],[0 0 1 1 0]);
  wires:
  poslin/1 -> "a{3}"/1
  netsum/1 -> poslin/1
  "b{3}"/1 -> netsum/2
  "LW{3,2}"/1("lz{3,2}") -> netsum/1
  Delays 1/1("pd{3,2}") -> "LW{3,2}"/1("ad{3,2}")
  "a{2} "/1 -> Delays 1/1("p{3}")

== system AcasXuClosedLoop_all_comp/networks/Networks_m_6/AcasXu_5_6/Layer 4 ==
  "a{3} " : Inport  PortDimensions=50
      mask Display: e = 0.06;c1 = [120 230 180]/255;c2 = ([120 230 180]+0)/(256+128);c3 = ([120 230 180]+128)/(256+128);patch([0 1 1 0],[0 0 1 1],c1);patch([0 1 1 1-e 1-e 0+e],[0 0 1 1-e 0+e 0+e],c2);patch([0 0 1 1-e 0+e 0+e],[0 1 1 1-e 1-e 0+e],c3);plot([0 1 1 0 0],[0 0 1 1 0]);
  Delays 1 : Subsystem
      mask Display: e = 0.06;c1 = [120 150 230]/255;c2 = ([120 150 230]+0)/383;c3 = ([120 150 230]+128)/383;patch([0 1 1 0],[0 0 1 1],c1);patch([0 1 1 1-e 1-e 0+e],[0 0 1 1-e 0+e 0+e],c2);patch([0 0 1 1-e 0+e 0+e],[0 1 1 1-e 1-e 0+e],c3);plot([0 1 1 0 0],[0 0 1 1 0]);disp('0');
  "LW{4,3}" : Subsystem
      mask Display: e = 0.06;c1 = [120 150 230]/255;c2 = ([120 150 230]+0)/383;c3 = ([120 150 230]+128)/383;patch([0 1 1 0],[0 0 1 1],c1);patch([0 1 1 1-e 1-e 0+e],[0 0 1 1-e 0+e 0+e],c2);patch([0 0 1 1-e 0+e 0+e],[0 1 1 1-e 1-e 0+e],c3);plot([0 1 1 0 0],[0 0 1 1 0]);disp('W');
  "b{4}" : Constant  Value=[-0.2018000000000000071498362785860081203281879425048828125;-1.1494899999999999007371798143140040338039398193359375;-1.0127200000000000645883346805931068956851959228515625;-2.06735999999999986442844601697288453578948974609375;-3.251889999999999947277729006600566208362579345703125;-0.52934099999999995045385503544821403920650482177734375;1.6736899999999999000266370785539038479328155517578125;-0.3308429999999999981952214511693455278873443603515625;-2.07218999999999997640998117276467382907867431640625;-0.77769600000000005390887736211880110204219818115234375;2.18780999999999981042719809920527040958404541015625;0.59206199999999997718447275474318303167819976806640625;-0.2132709999999999883613099882495589554309844970703125;0.57238599999999995038280076187220402061939239501953125;1.516950000000000020605739337042905390262603759765625;-0.424431999999999975958786535557010211050510406494140625;-0.9205710000000000281517031908151693642139434814453125;-0.63217599999999996018829051536158658564090728759765625;2.070320000000000160156332640326581895351409912109375;-1.35799000000000003041122909053228795528411865234375;-1.4100099999999999855759824640699662268161773681640625;-1.29821000000000008611777957412414252758026123046875;-1.190209999999999990194510246510617434978485107421875;0.4504610000000000002984279490192420780658721923828125;-1.9756400000000000627409235676168464124202728271484375;1.5180100000000000814992517916834913194179534912109375;0.7483969999999999789253024573554284870624542236328125;1.572459999999999968878228173707611858844757080078125;-0.01031089999999999957946972273248320561833679676055908203125;0.78002599999999999713651277488679625093936920166015625;0.437012000000000011556977597138029523193836212158203125;-2.18888999999999978030018610297702252864837646484375;-0.92857199999999995299049260211177170276641845703125;-1.5090399999999999369748593380791135132312774658203125;-1.68897999999999992581933838664554059505462646484375;-1.492869999999999919282345217652618885040283203125;1.9262200000000000432720526077901013195514678955078125;-1.78662000000000009691802915767766535282135009765625;-1.6711199999999999388222704510553739964962005615234375;0.2426089999999999913260495532085769809782505035400390625;-1.3017000000000000792255150372511707246303558349609375;0.496904999999999985593746032463968731462955474853515625;0.93028100000000002456346237522666342556476593017578125;-0.70829799999999998316724258984322659671306610107421875;-0.2083129999999999981685760985783417709171772003173828125;-0.34429399999999998893684960421524010598659515380859375;-1.4720299999999999496225200346088968217372894287109375;-0.6600230000000000263327137872693128883838653564453125;-1.06519999999999992468247000942938029766082763671875;-0.60161900000000001487165945945889689028263092041015625]
      mask Display: e = 0.06;c1 = [120 150 230]/255;c2 = ([120 150 230]+0)/383;c3 = ([120 150 230]+128)/383;patch([0 1 1 0],[0 0 1 1],c1);patch([0 1 1 1-e 1-e 0+e],[0 0 1 1-e 0+e 0+e],c2);patch([0 0 1 1-e 0+e 0+e],[0 1 1 1-e 1-e 0+e],c3);plot([0 1 1 0 0],[0 0 1 1 0]);disp('b');
  netsum : Sum  Inputs=2  SaturateOnIntegerOverflow=on  InputSameDT=on  OutDataTypeStr=Inherit: Same as first input
      mask Display: e = 0.06;c1 = [120 150 230]/255;c2 = ([120 150 230]+0)/383;c3 = ([120 150 230]+128)/383;patch([0 1 1 0],[0 0 1 1],c1);patch([0 1 1 1-e 1-e 0+e],[0 0 1 1-e 0+e 0+e],c2);patch([0 0 1 1-e 0+e 0+e],[0 1 1 1-e 1-e 0+e],c3);plot([0 1 1 0 0],[0 0 1 1 0]);disp('+');
  poslin : neural/Transfer Functions/poslin
      settings: SourceProductName=Deep Learning Toolbox  SourceProductBaseCode=NN
  "a{4}" : Outport  InitialOutput=[0;0;0;0;0;0;0;0;0;0;0;0;0;0;0;0;0;0;0;0;0;0;0;0;0;0;0;0;0;0;0;0;0;0;0;0;0;0;0;0;0;0;0;0;0;0;0;0;0;0]
      mask Display: e = 0.06;c1 = [120 230 180]/255;c2 = ([120 230 180]+0)/(256+128);c3 = ([120 230 180]+128)/(256+128);patch([0 1 1 0],[0 0 1 1],c1);patch([0 1 1 1-e 1-e 0+e],[0 0 1 1-e 0+e 0+e],c2);patch([0 0 1 1-e 0+e 0+e],[0 1 1 1-e 1-e 0+e],c3);plot([0 1 1 0 0],[0 0 1 1 0]);
  wires:
  poslin/1 -> "a{4}"/1
  netsum/1 -> poslin/1
  "b{4}"/1 -> netsum/2
  "LW{4,3}"/1("lz{4,3}") -> netsum/1
  Delays 1/1("pd{4,3}") -> "LW{4,3}"/1("ad{4,3}")
  "a{3} "/1 -> Delays 1/1("p{4}")
